simulink model "TosAeb"  (R2021a)
settings: Solver=FixedStepDiscrete  StartTime=0.0  StopTime=inf  FixedStep=0.01  RelTol=1e-3
undefined symbols (not defined in the file or in its folder): ACCModSts, AccSts1, AEB_ObjectMovingOrientation, AEB_ObjectMovingState, CN_BTCActive, CN_CurrentGearR, CN_CurrentGearR_DHT, CN_PTCActive, CN_VDCActive, CN_VSC_EspOff, CN_VSC_FCW_AEB_Error, CN_VSC_FCW_AEB_NoDispay, CN_VSC_FCW_AEB_PerfmDgdtn, CN_VSC_FCW_AEB_TempUnAvlbl, CN_VSC_VehStandstill, CNaccelAvoidMax, CNaccelAvoidMin, CNbrakePedalPress, CNcomfortStrLeftAccelMax, CNcomfortStrLeftAccelMin, CNcomfortStrRightAccelMax, CNcomfortStrRightAccelMin, CNDrvDoorOpen, CNDrvModeEco, CNDrvModeNorm, CNDrvModeOther, CNDrvModeSport, CNDrvSBRreminder, CNNull, CNobjInvalidID, CNPassDoorOpen, CNPassSBRreminder, CNPEBSAccelNegInf, CNPEBSAccelNull, CNPEBSTimeInf, CNPEBSTimeNull, CNSTOPMAX, CNSTOPNULL, CNsubjWidth, CNSwtOFF, CNSwtON, CNTTCMAX, CNTTCNULL, CNVehConfigAEBCycNone, CNVehConfigAEBNone, CNVehConfigAEBPedNone, CNVehConfigFCWNone, CNVehConfigJANone, CollisionSeverity, FALSE, FCWSnvty, JACycObjCalcInfoDefaultParams, JAPedObjCalcInfoDefaultParams, JAVehObjCalcInfoDefaultParams, latCycObjCalcInfoDefaultParams, latPedObjCalcInfoDefaultParams, longObjCalcInfoDefaultParams, ModSts, movingStatus, ObjectType ... (+1112)
libraries: simulink x3031, AEBSFunctionLib x50, Ta_Library x33, AEBSStateMachineLib x23, Mylibrary x12, simulink_extras x7, ObjSelect_lib x3, dspstat3 x2
(part 19 of 102)

== system TosAeb/FcwAebJa_Step_sys/ALG_AEBSystem/ALG_AEBController/AEB_VehicleStateCalcApp/VehMovingStateJudge/EngStateSuppFlg_HCU1 ==
  note: HCU_PowertrainSts:
      | 0x0:Sleep
      | 0x1:Initialize Mode
      | 0x2:power up
      | 0x3:power down
      | 0x4:Home
      | 0x5:run
      | 0x6:Charging
      | 0x7:Cellphone AC
      | 0x8:Limphome
      | 0x9:Emergency Mode
      | 0xA:V2L
      | 0xB:Prepare Sleep
      | 0xC:ready
      | 0xD:LV Charging
      | 0xE:LT Charging
      | 0xF:IL heating
      | 0x10:Thermal run away
      | 0x11:End of line
      | 0x12:BattTempKeep
      | 0x13-0x1F:Reserved
  note: HCU_Start_StopSts:
      | 0x0:non-start/stop mode
      | 0x1:Engine standby
      | 0x2:Normal operation
      | 0x3:Engine stop preparation
      | 0x4:Running down
      | 0x5:Engine stopped
      | 0x6:Cranking
      | 0x7:Starting
      | 0x8:Transmit torque
      | 0x9:Reserved
  note: EngState:
      | 0x0:Stopped
      | 0x1:Cranking
      | 0x2:Running
      | 0x3:Stalling
  EngState : Inport
  HCU_PowertrainSts : Inport  Port=2
  HCU_Start_StopSts : Inport  Port=3
  subjVel : Inport  Port=4
  Compare To Constant : simulink/Logic and Bit Operations/Compare To Constant  relop===  const=uint8(5)  OutDataTypeStr=boolean
  Compare To Constant1 : simulink/Logic and Bit Operations/Compare To Constant  relop===  const=uint8(5)  OutDataTypeStr=boolean
  Compare To Constant2 : simulink/Logic and Bit Operations/Compare To Constant  relop===  const=uint8(0)  OutDataTypeStr=boolean
  Compare To Constant4 : simulink/Logic and Bit Operations/Compare To Constant  relop=<  const=P_AEB_vehStateCalc_SubjVelThres4HCU_EngCond  OutDataTypeStr=boolean
  Constant : Constant  Value=FALSE
  Constant1 : Constant  Value=TRUE
  Logical Operator : Logic  Inputs=3  AllPortsSameDT=off  OutDataTypeStr=boolean
  Logical Operator1 : Logic  Operator=OR  AllPortsSameDT=off  OutDataTypeStr=boolean
  Switch : Switch  Criteria=u2 ~= 0
  EngStateSuppFlg : Outport
  wires:
  subjVel/1 -> Compare To Constant4/1
  Logical Operator1/1 -> Switch/2
  Logical Operator/1 -> Logical Operator1/2
  Compare To Constant4/1 -> Logical Operator/3
  Compare To Constant2/1 -> Logical Operator/2
  Compare To Constant1/1 -> Logical Operator/1
  EngState/1 -> Compare To Constant2/1
  HCU_Start_StopSts/1 -> Compare To Constant1/1
  Constant1/1 -> Switch/3
  Compare To Constant/1 -> Logical Operator1/1
  Constant/1 -> Switch/1
  HCU_PowertrainSts/1 -> Compare To Constant/1
  Switch/1 -> EngStateSuppFlg/1

== system TosAeb/FcwAebJa_Step_sys/ALG_AEBSystem/ALG_AEBController/AEB_VehicleStateCalcApp/VehMovingStateJudge/Subsystem1 ==
  note: 0x0:Select lever in position "P"
      | 0x1:Reserved
      | 0x2:Reserved
      | 0x3:Reserved
      | 0x4:Reserved
      | 0x5:Select lever in position "D"
      | 0x6:Select lever in position "N"
      | 0x7:Select lever in position "R"
      | 0x08-0xD:Reserved
      | 0xE:Intermediate position
      | 0xF:Invalid
  note: P_Global_VehiclePowerType
      | 0-V71混动；1-V61混动；
  CURRENT_GEAR : Inport
  HCU_GearSts : Inport  Port=2
  Compare To Constant : simulink/Logic and Bit Operations/Compare To Constant  relop===  const=CN_CurrentGearR  OutDataTypeStr=boolean
  Compare To Constant1 : simulink/Logic and Bit Operations/Compare To Constant  relop===  const=CN_CurrentGearR_DHT  OutDataTypeStr=boolean
  Constant : Constant  Value=P_Global_VehiclePowerType
  Multiport Switch : MultiPortSwitch  DataPortOrder=Specify indices  DataPortIndices={1,0}
  TCU_OperatingStateSuppFlg : Outport
  wires:
  Compare To Constant1/1 -> Multiport Switch/2  "TCUOperatingStateSuppFlg"
  Compare To Constant/1 -> Multiport Switch/3  "TCUOperatingStateSuppFlg"
  Multiport Switch/1 -> TCU_OperatingStateSuppFlg/1
  HCU_GearSts/1 -> Compare To Constant1/1
  Constant/1 -> Multiport Switch/1
  CURRENT_GEAR/1 -> Compare To Constant/1

== system TosAeb/FcwAebJa_Step_sys/ALG_AEBSystem/ALG_AEBController/AEB_VehicleStateCalcApp/VehSuppAborCondtionCalc/ABASuppAborConditonCalc ==
  AEB_VehResp : Inport
  FCW_AEB_VehFctSts : Inport  Port=2
  vehUnstableStateFlg : Inport  Port=3
  vehAssociatedSysStateSuppCond : Inport  Port=4
  "vehConfigAEB " : Inport  Port=5
  blockTimeBrakeReady : Inport  Port=6
  Bus Creator : BusCreator  Inputs=6
  Bus Selector : BusSelector  OutputSignals=AEB_VehResp,FCW_AEB_VehFctSts,vehUnstableSts,vehAssociatedSysStsSuppFlg,VehicleConfigAEB,blockTimeBrakeReady
  Bus Selector1 : BusSelector  OutputSignals=AEB_VehResp,FCW_AEB_VehFctSts
  VehStateCalcABAAborCalc : Subsystem
  VehStateCalcABAtSuppCalc : Subsystem
  VSCvehABASuppCond : Outport
  VSCvehABAAborCond : Outport  Port=2
  wires:
  VehStateCalcABAAborCalc/1(VSCvehABAAborCond) -> VSCvehABAAborCond/1
  VehStateCalcABAtSuppCalc/1(VSCvehABASuppCond) -> VSCvehABASuppCond/1
  Bus Selector1/2 -> VehStateCalcABAAborCalc/2(FCW_AEB_VehFctSts)  "<FCW_AEB_VehFctSts>"
  Bus Selector1/1 -> VehStateCalcABAAborCalc/1(AEB_VehResp)  "<AEB_VehResp>"
  Bus Selector/6 -> VehStateCalcABAtSuppCalc/6(blockTimeBrakeReady)  "<blockTimeBrakeReady>"
  Bus Selector/5 -> VehStateCalcABAtSuppCalc/5("vehConfigAEB ")  "<VehicleConfigAEB>"
  Bus Selector/4 -> VehStateCalcABAtSuppCalc/4(vehAssociatedSysStateSuppCond)  "<vehAssociatedSysStsSuppFlg>"
  Bus Selector/3 -> VehStateCalcABAtSuppCalc/3(vehUnstableStateFlg)  "<vehUnstableSts>"
  Bus Selector/2 -> VehStateCalcABAtSuppCalc/2(FCW_AEB_VehFctSts)  "<FCW_AEB_VehFctSts>"
  Bus Selector/1 -> VehStateCalcABAtSuppCalc/1(AEB_VehResp)  "<AEB_VehResp>"
  Bus Creator/1 -> Bus Selector1/1, Bus Selector/1
  AEB_VehResp/1 -> Bus Creator/1
  FCW_AEB_VehFctSts/1 -> Bus Creator/2
  blockTimeBrakeReady/1 -> Bus Creator/6
  vehUnstableStateFlg/1 -> Bus Creator/3
  "vehConfigAEB "/1 -> Bus Creator/5
  vehAssociatedSysStateSuppCond/1 -> Bus Creator/4

== system TosAeb/FcwAebJa_Step_sys/ALG_AEBSystem/ALG_AEBController/AEB_VehicleStateCalcApp/VehSuppAborCondtionCalc/AEBfullSuppAborConditonCalc ==
  VSCvehAEB_VehResp : Inport
  FCW_AEB_VehFctSts : Inport  Port=2
  vehUnstableStateFlg : Inport  Port=3
  vehAssociatedSysStateSuppCond : Inport  Port=4
  "vehConfigAEB " : Inport  Port=5
  blockTimeBrake : Inport  Port=6
  Bus Creator : BusCreator  Inputs=6
  Bus Selector : BusSelector  OutputSignals=AEB_VehResp,FCW_AEB_VehFctSts,vehUnstableSts,vehAssociatedSysStsSuppFlg,VehicleConfigAEB,blockTimeBrake
  Bus Selector1 : BusSelector  OutputSignals=AEB_VehResp,FCW_AEB_VehFctSts,vehAssociatedSysStsSuppFlg,vehUnstableSts
  VehStateCalcAEBfullAborCalc : Subsystem
  VehStateCalcAEBfullSuppCalc : Subsystem
  VSCvehAEBfullSuppCond : Outport
  VSCvehAEBfullAborCond : Outport  Port=2
  wires:
  VehStateCalcAEBfullAborCalc/1(VSCvehAEBfullAborCond) -> VSCvehAEBfullAborCond/1
  VehStateCalcAEBfullSuppCalc/1(VSCvehAEBfullSuppCond) -> VSCvehAEBfullSuppCond/1
  Bus Selector1/4 -> VehStateCalcAEBfullAborCalc/4(vehUnstableStateFlg)  "<vehUnstableSts>"
  Bus Selector1/3 -> VehStateCalcAEBfullAborCalc/3(vehAssociatedSysStateSuppCond)  "<vehAssociatedSysStsSuppFlg>"
  Bus Selector1/2 -> VehStateCalcAEBfullAborCalc/2(FCW_AEB_VehFctSts)  "<FCW_AEB_VehFctSts>"
  Bus Selector1/1 -> VehStateCalcAEBfullAborCalc/1(AEB_VehResp)  "<AEB_VehResp>"
  Bus Selector/6 -> VehStateCalcAEBfullSuppCalc/6(blockTimeBrake)  "<blockTimeBrake>"
  Bus Selector/5 -> VehStateCalcAEBfullSuppCalc/5("vehConfigAEB ")  "<VehicleConfigAEB>"
  Bus Selector/4 -> VehStateCalcAEBfullSuppCalc/4(vehAssociatedSysStateSuppCond)  "<vehAssociatedSysStsSuppFlg>"
  Bus Selector/3 -> VehStateCalcAEBfullSuppCalc/3(vehUnstableStateFlg)  "<vehUnstableSts>"
  Bus Selector/2 -> VehStateCalcAEBfullSuppCalc/2(FCW_AEB_VehFctSts)  "<FCW_AEB_VehFctSts>"
  Bus Selector/1 -> VehStateCalcAEBfullSuppCalc/1(AEB_VehResp)  "<AEB_VehResp>"
  Bus Creator/1 -> Bus Selector/1, Bus Selector1/1
  VSCvehAEB_VehResp/1 -> Bus Creator/1
  blockTimeBrake/1 -> Bus Creator/6
  FCW_AEB_VehFctSts/1 -> Bus Creator/2
  "vehConfigAEB "/1 -> Bus Creator/5
  vehUnstableStateFlg/1 -> Bus Creator/3
  vehAssociatedSysStateSuppCond/1 -> Bus Creator/4

== system TosAeb/FcwAebJa_Step_sys/ALG_AEBSystem/ALG_AEBController/AEB_VehicleStateCalcApp/VehSuppAborCondtionCalc/AEBpartSuppAborConditonCalc ==
  AEB_VehResp : Inport
  FCW_AEB_VehFctSts : Inport  Port=2
  vehUnstableStateFlg : Inport  Port=3
  vehAssociatedSysStateSuppCond : Inport  Port=4
  "vehConfigAEB " : Inport  Port=5
  blockTimeBrake : Inport  Port=6
  Bus Creator : BusCreator  Inputs=6
  Bus Selector : BusSelector  OutputSignals=AEB_VehResp,FCW_AEB_VehFctSts,vehUnstableSts,vehAssociatedSysStsSuppFlg,VehicleConfigAEB,blockTimeBrake
  Bus Selector1 : BusSelector  OutputSignals=AEB_VehResp,FCW_AEB_VehFctSts,vehAssociatedSysStsSuppFlg,vehUnstableSts
  VehStateCalcAEBpartAborCalc : Subsystem
  VehStateCalcAEBpartSuppCalc : Subsystem
  VSCvehAEBpartSuppCond : Outport
  VSCvehAEBpartAborCond : Outport  Port=2
  wires:
  VehStateCalcAEBpartAborCalc/1(VSCvehAEBpartAborCond) -> VSCvehAEBpartAborCond/1
  VehStateCalcAEBpartSuppCalc/1(VSCvehAEBpartSuppCond) -> VSCvehAEBpartSuppCond/1
  Bus Selector1/4 -> VehStateCalcAEBpartAborCalc/4(vehUnstableStateFlg)  "<vehUnstableSts>"
  Bus Selector1/3 -> VehStateCalcAEBpartAborCalc/3(vehAssociatedSysStateSuppCond)  "<vehAssociatedSysStsSuppFlg>"
  Bus Selector1/2 -> VehStateCalcAEBpartAborCalc/2(FCW_AEB_VehFctSts)  "<FCW_AEB_VehFctSts>"
  Bus Selector1/1 -> VehStateCalcAEBpartAborCalc/1(AEB_VehResp)  "<AEB_VehResp>"
  Bus Selector/1 -> VehStateCalcAEBpartSuppCalc/1(AEB_VehResp)  "<AEB_VehResp>"
  Bus Selector/6 -> VehStateCalcAEBpartSuppCalc/6(blockTimeBrake)  "<blockTimeBrake>"
  Bus Selector/2 -> VehStateCalcAEBpartSuppCalc/2(FCW_AEB_VehFctSts)  "<FCW_AEB_VehFctSts>"
  Bus Selector/5 -> VehStateCalcAEBpartSuppCalc/5("vehConfigAEB ")  "<VehicleConfigAEB>"
  Bus Selector/3 -> VehStateCalcAEBpartSuppCalc/3(vehUnstableStateFlg)  "<vehUnstableSts>"
  Bus Selector/4 -> VehStateCalcAEBpartSuppCalc/4(vehAssociatedSysStateSuppCond)  "<vehAssociatedSysStsSuppFlg>"
  Bus Creator/1 -> Bus Selector/1, Bus Selector1/1
  blockTimeBrake/1 -> Bus Creator/6
  "vehConfigAEB "/1 -> Bus Creator/5
  vehAssociatedSysStateSuppCond/1 -> Bus Creator/4
  vehUnstableStateFlg/1 -> Bus Creator/3
  FCW_AEB_VehFctSts/1 -> Bus Creator/2
  AEB_VehResp/1 -> Bus Creator/1

== system TosAeb/FcwAebJa_Step_sys/ALG_AEBSystem/ALG_AEBController/AEB_VehicleStateCalcApp/VehSuppAborCondtionCalc/AcuteWarningSuppAborConditonCalc ==
  AEB_VehResp : Inport
  FCW_AEB_VehFctSts : Inport  Port=2
  vehUnstableStateFlg : Inport  Port=3
  vehAssociatedSysStateSuppCond : Inport  Port=4
  "vehConfigFCW " : Inport  Port=5
  blockTimeWarning : Inport  Port=6
  Bus Creator : BusCreator  Inputs=6
  Bus Selector : BusSelector  OutputSignals=AEB_VehResp,FCW_AEB_VehFctSts,vehUnstableSts,vehAssociatedSysStsSuppFlg,VehicleConfigFCW,blockTimeWarning
  Bus Selector1 : BusSelector  OutputSignals=AEB_VehResp,FCW_AEB_VehFctSts,vehAssociatedSysStsSuppFlg
  VehStateCalcAcuteWarningAborCalc : Subsystem
  VehStateCalcAcuteWarningSuppCalc : Subsystem
  VSCvehAcuteWarningSuppCond : Outport
  VSCvehAcuteWarningAborCond : Outport  Port=2
  wires:
  VehStateCalcAcuteWarningAborCalc/1(VSCvehAcuteWarningAborCond) -> VSCvehAcuteWarningAborCond/1
  VehStateCalcAcuteWarningSuppCalc/1(VSCvehAcuteWarningSuppCond) -> VSCvehAcuteWarningSuppCond/1
  Bus Selector1/3 -> VehStateCalcAcuteWarningAborCalc/3(vehAssociatedSysStateSuppCond)  "<vehAssociatedSysStsSuppFlg>"
  Bus Selector1/1 -> VehStateCalcAcuteWarningAborCalc/1(AEB_VehResp)  "<AEB_VehResp>"
  Bus Selector1/2 -> VehStateCalcAcuteWarningAborCalc/2(FCW_AEB_VehFctSts)  "<FCW_AEB_VehFctSts>"
  Bus Selector/1 -> VehStateCalcAcuteWarningSuppCalc/1(AEB_VehResp)  "<AEB_VehResp>"
  Bus Selector/6 -> VehStateCalcAcuteWarningSuppCalc/6(blockTimeWarning)  "<blockTimeWarning>"
  Bus Selector/2 -> VehStateCalcAcuteWarningSuppCalc/2(FCW_AEB_VehFctSts)  "<FCW_AEB_VehFctSts>"
  Bus Selector/5 -> VehStateCalcAcuteWarningSuppCalc/5("vehConfigFCW ")  "<VehicleConfigFCW>"
  Bus Selector/3 -> VehStateCalcAcuteWarningSuppCalc/3(vehUnstableStateFlg)  "<vehUnstableSts>"
  Bus Selector/4 -> VehStateCalcAcuteWarningSuppCalc/4(vehAssociatedSysStateSuppCond)  "<vehAssociatedSysStsSuppFlg>"
  Bus Creator/1 -> Bus Selector1/1, Bus Selector/1
  AEB_VehResp/1 -> Bus Creator/1
  FCW_AEB_VehFctSts/1 -> Bus Creator/2
  vehUnstableStateFlg/1 -> Bus Creator/3
  vehAssociatedSysStateSuppCond/1 -> Bus Creator/4
  "vehConfigFCW "/1 -> Bus Creator/5
  blockTimeWarning/1 -> Bus Creator/6

== system TosAeb/FcwAebJa_Step_sys/ALG_AEBSystem/ALG_AEBController/AEB_VehicleStateCalcApp/VehSuppAborCondtionCalc/EBASuppAborConditonCalc ==
  AEB_VehResp : Inport
  FCW_AEB_VehFctSts : Inport  Port=2
  vehUnstableStateFlg : Inport  Port=3
  vehAssociatedSysStateSuppCond : Inport  Port=4
  "vehConfigAEB " : Inport  Port=5
  blockTimeBrake : Inport  Port=6
  Bus Creator : BusCreator  Inputs=6
  Bus Selector : BusSelector  OutputSignals=AEB_VehResp,FCW_AEB_VehFctSts,vehUnstableSts,vehAssociatedSysStsSuppFlg,VehicleConfigAEB,blockTimeBrake
  Bus Selector1 : BusSelector  OutputSignals=AEB_VehResp,FCW_AEB_VehFctSts,vehAssociatedSysStsSuppFlg
  VehStateCalcEBAAborCalc : Subsystem
  VehStateCalcEBASuppCalc : Subsystem
  VSCvehEBASuppCond : Outport
  VSCvehEBAAborCond : Outport  Port=2
  wires:
  VehStateCalcEBAAborCalc/1(VSCvehEBAAborCond) -> VSCvehEBAAborCond/1
  VehStateCalcEBASuppCalc/1(VSCvehEBASuppCond) -> VSCvehEBASuppCond/1
  Bus Selector1/3 -> VehStateCalcEBAAborCalc/3(vehAssociatedSysStateSuppCond)  "<vehAssociatedSysStsSuppFlg>"
  Bus Selector1/1 -> VehStateCalcEBAAborCalc/1(AEB_VehResp)  "<AEB_VehResp>"
  Bus Selector1/2 -> VehStateCalcEBAAborCalc/2(FCW_AEB_VehFctSts)  "<FCW_AEB_VehFctSts>"
  Bus Selector/1 -> VehStateCalcEBASuppCalc/1(AEB_VehResp)  "<AEB_VehResp>"
  Bus Selector/6 -> VehStateCalcEBASuppCalc/6(blockTimeBrake)  "<blockTimeBrake>"
  Bus Selector/2 -> VehStateCalcEBASuppCalc/2(FCW_AEB_VehFctSts)  "<FCW_AEB_VehFctSts>"
  Bus Selector/5 -> VehStateCalcEBASuppCalc/5("vehConfigAEB ")  "<VehicleConfigAEB>"
  Bus Selector/3 -> VehStateCalcEBASuppCalc/3(vehUnstableStateFlg)  "<vehUnstableSts>"
  Bus Selector/4 -> VehStateCalcEBASuppCalc/4(vehAssociatedSysStateSuppCond)  "<vehAssociatedSysStsSuppFlg>"
  Bus Creator/1 -> Bus Selector1/1, Bus Selector/1
  AEB_VehResp/1 -> Bus Creator/1
  FCW_AEB_VehFctSts/1 -> Bus Creator/2
  blockTimeBrake/1 -> Bus Creator/6
  vehUnstableStateFlg/1 -> Bus Creator/3
  "vehConfigAEB "/1 -> Bus Creator/5
  vehAssociatedSysStateSuppCond/1 -> Bus Creator/4

== system TosAeb/FcwAebJa_Step_sys/ALG_AEBSystem/ALG_AEBController/AEB_VehicleStateCalcApp/VehSuppAborCondtionCalc/LatentWarningSuppAborConditonCalc ==
  AEB_VehResp : Inport
  FCW_AEB_VehFctSts : Inport  Port=2
  vehUnstableStateFlg : Inport  Port=3
  vehAssociatedSysStateSuppCond : Inport  Port=4
  "vehConfigFCW " : Inport  Port=5
  blockTimeWarning : Inport  Port=6
  Bus Creator : BusCreator  Inputs=6
  Bus Selector : BusSelector  OutputSignals=AEB_VehResp,FCW_AEB_VehFctSts,vehUnstableSts,vehAssociatedSysStsSuppFlg,VehicleConfigFCW,blockTimeWarning
  Bus Selector1 : BusSelector  OutputSignals=AEB_VehResp,FCW_AEB_VehFctSts,vehAssociatedSysStsSuppFlg
  VehStateCalcLatentWarningAborCalc : Subsystem
  VehStateCalcLatentWarningSuppCalc : Subsystem
  VSCvehLatentWarningSuppCond : Outport
  VSCvehLatentWarningAborCond : Outport  Port=2
  wires:
  VehStateCalcLatentWarningAborCalc/1(VSCvehLatentWarningAborCond) -> VSCvehLatentWarningAborCond/1
  VehStateCalcLatentWarningSuppCalc/1(VSCvehLatentWarningSuppCond) -> VSCvehLatentWarningSuppCond/1
  Bus Selector1/3 -> VehStateCalcLatentWarningAborCalc/3(vehAssociatedSysStateSuppCond)  "<vehAssociatedSysStsSuppFlg>"
  Bus Selector1/1 -> VehStateCalcLatentWarningAborCalc/1(AEB_VehResp)  "<AEB_VehResp>"
  Bus Selector1/2 -> VehStateCalcLatentWarningAborCalc/2(FCW_AEB_VehFctSts)  "<FCW_AEB_VehFctSts>"
  Bus Selector/1 -> VehStateCalcLatentWarningSuppCalc/1(AEB_VehResp)  "<AEB_VehResp>"
  Bus Selector/6 -> VehStateCalcLatentWarningSuppCalc/6(blockTimeWarning)  "<blockTimeWarning>"
  Bus Selector/2 -> VehStateCalcLatentWarningSuppCalc/2(FCW_AEB_VehFctSts)  "<FCW_AEB_VehFctSts>"
  Bus Selector/5 -> VehStateCalcLatentWarningSuppCalc/5("vehConfigFCW ")  "<VehicleConfigFCW>"
  Bus Selector/3 -> VehStateCalcLatentWarningSuppCalc/3(vehUnstableStateFlg)  "<vehUnstableSts>"
  Bus Selector/4 -> VehStateCalcLatentWarningSuppCalc/4(vehAssociatedSysStateSuppCond)  "<vehAssociatedSysStsSuppFlg>"
  Bus Creator/1 -> Bus Selector1/1, Bus Selector/1
  blockTimeWarning/1 -> Bus Creator/6
  "vehConfigFCW "/1 -> Bus Creator/5
  vehAssociatedSysStateSuppCond/1 -> Bus Creator/4
  vehUnstableStateFlg/1 -> Bus Creator/3
  FCW_AEB_VehFctSts/1 -> Bus Creator/2
  AEB_VehResp/1 -> Bus Creator/1

== system TosAeb/FcwAebJa_Step_sys/ALG_AEBSystem/ALG_AEBController/AEB_VehicleStateCalcApp/VehSuppAborCondtionCalc/PreWarningSuppAborConditonCalc ==
  AEB_VehResp : Inport
  FCW_AEB_VehFctSts : Inport  Port=2
  vehUnstableStateFlg : Inport  Port=3
  vehAssociatedSysSuppStateFlg : Inport  Port=4
  "vehConfigFCW " : Inport  Port=5
  blockTimeWarning : Inport  Port=6
  Bus Creator : BusCreator  Inputs=6
  Bus Selector : BusSelector  OutputSignals=AEB_VehResp,FCW_AEB_VehFctSts,vehUnstableSts,vehAssociatedSysStsSuppFlg,VehicleConfigFCW,blockTimeWarning
  Bus Selector1 : BusSelector  OutputSignals=AEB_VehResp,FCW_AEB_VehFctSts,vehAssociatedSysStsSuppFlg
  VehStateCalcPreWarningAborCalc : Subsystem
  VehStateCalcPreWarningSuppCalc : Subsystem
  VSCvehPreWarningSuppCond : Outport
  VSCvehPreWarningAborCond : Outport  Port=2
  wires:
  VehStateCalcPreWarningAborCalc/1(VSCvehPreWarningAborCond) -> VSCvehPreWarningAborCond/1
  VehStateCalcPreWarningSuppCalc/1(VSCvehPreWarningSuppCond) -> VSCvehPreWarningSuppCond/1
  Bus Selector1/3 -> VehStateCalcPreWarningAborCalc/3(vehAssociatedSysStateSuppCond)  "<vehAssociatedSysStsSuppFlg>"
  Bus Selector1/2 -> VehStateCalcPreWarningAborCalc/2(FCW_AEB_VehFctSts)  "<FCW_AEB_VehFctSts>"
  Bus Selector1/1 -> VehStateCalcPreWarningAborCalc/1(AEB_VehResp)  "<AEB_VehResp>"
  Bus Selector/1 -> VehStateCalcPreWarningSuppCalc/1(AEB_VehResp)  "<AEB_VehResp>"
  Bus Selector/6 -> VehStateCalcPreWarningSuppCalc/6(blockTimeWarning)  "<blockTimeWarning>"
  Bus Selector/2 -> VehStateCalcPreWarningSuppCalc/2(FCW_AEB_VehFctSts)  "<FCW_AEB_VehFctSts>"
  Bus Selector/5 -> VehStateCalcPreWarningSuppCalc/5("vehConfigFCW ")  "<VehicleConfigFCW>"
  Bus Selector/3 -> VehStateCalcPreWarningSuppCalc/3(vehUnstableStateFlg)  "<vehUnstableSts>"
  Bus Selector/4 -> VehStateCalcPreWarningSuppCalc/4(vehAssociatedSysStateSuppCond)  "<vehAssociatedSysStsSuppFlg>"
  Bus Creator/1 -> Bus Selector1/1, Bus Selector/1
  AEB_VehResp/1 -> Bus Creator/1
  FCW_AEB_VehFctSts/1 -> Bus Creator/2
  vehUnstableStateFlg/1 -> Bus Creator/3
  vehAssociatedSysSuppStateFlg/1 -> Bus Creator/4
  blockTimeWarning/1 -> Bus Creator/6
  "vehConfigFCW "/1 -> Bus Creator/5

== system TosAeb/FcwAebJa_Step_sys/ALG_AEBSystem/ALG_AEBController/AEB_VehicleStateCalcApp/VehSuppAborCondtionCalc/PrefillSuppAborConditonCalc ==
  AEB_VehResp : Inport
  FCW_AEB_VehFctSts : Inport  Port=2
  vehUnstableStateFlg : Inport  Port=3
  vehAssociatedSysStateSuppCond : Inport  Port=4
  "vehConfigAEB " : Inport  Port=5
  blockTimeBrakeReady : Inport  Port=6
  Bus Creator : BusCreator  Inputs=6
  Bus Selector : BusSelector  OutputSignals=AEB_VehResp,FCW_AEB_VehFctSts,vehUnstableSts,vehAssociatedSysStsSuppFlg,VehicleConfigAEB,blockTimeBrakeReady
  Bus Selector1 : BusSelector  OutputSignals=AEB_VehResp,FCW_AEB_VehFctSts,vehUnstableSts,vehAssociatedSysStsSuppFlg
  VehStateCalcPrefillAborCalc : Subsystem
  VehStateCalcPrefillSuppCalc : Subsystem
  VSCvehPrefillSuppCond : Outport
  VSCvehPrefillAborCond : Outport  Port=2
  wires:
  VehStateCalcPrefillAborCalc/1(VSCvehPrefillAborCond) -> VSCvehPrefillAborCond/1
  VehStateCalcPrefillSuppCalc/1(VSCvehPrefillSuppCond) -> VSCvehPrefillSuppCond/1
  Bus Selector1/4 -> VehStateCalcPrefillAborCalc/4(vehAssociatedSysStateSuppCond)  "<vehAssociatedSysStsSuppFlg>"
  Bus Selector1/3 -> VehStateCalcPrefillAborCalc/3(vehUnstableStateFlg)  "<vehUnstableSts>"
  Bus Selector1/2 -> VehStateCalcPrefillAborCalc/2(FCW_AEB_VehFctSts)  "<FCW_AEB_VehFctSts>"
  Bus Selector1/1 -> VehStateCalcPrefillAborCalc/1(AEB_VehResp)  "<AEB_VehResp>"
  Bus Selector/6 -> VehStateCalcPrefillSuppCalc/6(blockTimeBrakeReady)  "<blockTimeBrakeReady>"
  Bus Selector/1 -> VehStateCalcPrefillSuppCalc/1(AEB_VehResp)  "<AEB_VehResp>"
  Bus Selector/5 -> VehStateCalcPrefillSuppCalc/5("vehConfigAEB ")  "<VehicleConfigAEB>"
  Bus Selector/2 -> VehStateCalcPrefillSuppCalc/2(FCW_AEB_VehFctSts)  "<FCW_AEB_VehFctSts>"
  Bus Selector/4 -> VehStateCalcPrefillSuppCalc/4(vehAssociatedSysStateSuppCond)  "<vehAssociatedSysStsSuppFlg>"
  Bus Selector/3 -> VehStateCalcPrefillSuppCalc/3(vehUnstableStateFlg)  "<vehUnstableSts>"
  Bus Creator/1 -> Bus Selector/1, Bus Selector1/1
  AEB_VehResp/1 -> Bus Creator/1
  blockTimeBrakeReady/1 -> Bus Creator/6
  FCW_AEB_VehFctSts/1 -> Bus Creator/2
  "vehConfigAEB "/1 -> Bus Creator/5
  vehUnstableStateFlg/1 -> Bus Creator/3
  vehAssociatedSysStateSuppCond/1 -> Bus Creator/4

== system TosAeb/FcwAebJa_Step_sys/ALG_AEBSystem/ALG_AEBInput/JaObjInfoMap/JaObjInfoMap/MovingStatus_Subsystem ==
  Alg_MovingStatus : Inport
  InputMovingStatus : Subsystem  TreatAsAtomicUnit=on
  MovingStatusSwitch : Stateflow chart  TreatAsAtomicUnit=on
  MovingStatusOut : Outport
  wires:
  Alg_MovingStatus/1 -> InputMovingStatus/1(MovingStatus)
  MovingStatusSwitch/1(MovingStatus) -> MovingStatusOut/1
  InputMovingStatus/1(Input_Unknown) -> MovingStatusSwitch/1(Input_Unknown)
  InputMovingStatus/2(Input_Stationary) -> MovingStatusSwitch/2(Input_Stationary)
  InputMovingStatus/3(Input_Stopped) -> MovingStatusSwitch/3(Input_Stopped)
  InputMovingStatus/4(Input_Moving) -> MovingStatusSwitch/4(Input_Moving)

== system TosAeb/FcwAebJa_Step_sys/ALG_AEBSystem/ALG_AEBInput/JaObjInfoMap/JaObjInfoMap/ObjType_Subsystem ==
  Alg_ObjType : Inport
  InputObjectType : Subsystem  TreatAsAtomicUnit=on
  ObjectType : Stateflow chart  TreatAsAtomicUnit=on
  ObjType : Outport
  wires:
  InputObjectType/4(Input_ObjMotor) -> ObjectType/4(ObjMotor)
  InputObjectType/6(Input_ObjGeneral) -> ObjectType/6(ObjGeneral)
  InputObjectType/3(Input_ObjPed) -> ObjectType/3(ObjPed)
  InputObjectType/7(Input_Unknown) -> ObjectType/7(Unknown)
  InputObjectType/5(Input_ObjBicycle) -> ObjectType/5(ObjBicycle)
  InputObjectType/2(Input_ObjTruck) -> ObjectType/2(ObjTruck)
  InputObjectType/1(Input_ObjCar) -> ObjectType/1(ObjCar)
  ObjectType/1(ObjType) -> ObjType/1
  Alg_ObjType/1 -> InputObjectType/1(u)

== system TosAeb/FcwAebJa_Step_sys/ALG_AEBSystem/ALG_AEBInput/JaObjInfoMap/JaObjInfoMap/Subsystem ==
  FR_ObjectType : Inport
  FR_ObjectMovingState : Inport  Port=2
  FR_ObjectMovingOrientation : Inport  Port=3
  Data Type Conversion : DataTypeConversion  OutDataTypeStr=Enum: AEB_ObjectMovingOrientation
  Data Type Conversion1 : DataTypeConversion  OutDataTypeStr=Enum: ObjectTypeCopy
  Data Type Conversion2 : DataTypeConversion  OutDataTypeStr=Enum: AEB_ObjectMovingState
  MovingStatus_Subsystem : Subsystem
  ObjType_Subsystem1 : Subsystem
  FR_ObjectTypeFilt : Outport
  FR_ObjectMovingStateFilt : Outport  Port=2
  FR_ObjectMovingOrientationFilt : Outport  Port=3
  wires:
  FR_ObjectType/1 -> ObjType_Subsystem1/1(Alg_ObjType)
  ObjType_Subsystem1/1(ObjType) -> Data Type Conversion1/1  "ObjectType"
  Data Type Conversion1/1 -> FR_ObjectTypeFilt/1  "ObjectType"
  MovingStatus_Subsystem/1(MovingStatusOut) -> Data Type Conversion2/1
  FR_ObjectMovingState/1 -> MovingStatus_Subsystem/1(Alg_MovingStatus)
  Data Type Conversion2/1 -> FR_ObjectMovingStateFilt/1
  FR_ObjectMovingOrientation/1 -> Data Type Conversion/1
  Data Type Conversion/1 -> FR_ObjectMovingOrientationFilt/1  "ObjectMovingOrientation"

== system TosAeb/FcwAebJa_Step_sys/ALG_AEBSystem/ALG_AEBInput/JaObjInfoMap/JaObjInfoMap/Subsystem1 ==
  FC_ObjectType : Inport
  FC_ObjectMovingState : Inport  Port=2
  FC_ObjectMovingOrientation : Inport  Port=3
  Data Type Conversion : DataTypeConversion  OutDataTypeStr=Enum: AEB_ObjectMovingOrientation
  Data Type Conversion1 : DataTypeConversion  OutDataTypeStr=Enum: ObjectTypeCopy
  Data Type Conversion2 : DataTypeConversion  OutDataTypeStr=Enum: AEB_ObjectMovingState
  MovingStatus_Subsystem : Subsystem
  ObjType_Subsystem1 : Subsystem
  FC_ObjectTypeFilt : Outport
  FC_ObjectMovingStateFilt : Outport  Port=2
  FC_ObjectMovingOrientationFilt : Outport  Port=3
  wires:
  FC_ObjectType/1 -> ObjType_Subsystem1/1(Alg_ObjType)
  ObjType_Subsystem1/1(ObjType) -> Data Type Conversion1/1  "ObjectType"
  Data Type Conversion1/1 -> FC_ObjectTypeFilt/1  "ObjectType"
  MovingStatus_Subsystem/1(MovingStatusOut) -> Data Type Conversion2/1
  FC_ObjectMovingState/1 -> MovingStatus_Subsystem/1(Alg_MovingStatus)
  Data Type Conversion2/1 -> FC_ObjectMovingStateFilt/1
  FC_ObjectMovingOrientation/1 -> Data Type Conversion/1
  Data Type Conversion/1 -> FC_ObjectMovingOrientationFilt/1  "ObjectMovingOrientation"

== system TosAeb/FcwAebJa_Step_sys/ALG_AEBSystem/ALG_AEBInput/LatObjInfoMap/latObjInfoMap/MovingStatus_Subsystem ==
  Alg_MovingStatus : Inport
  InputMovingStatus : Subsystem  TreatAsAtomicUnit=on
  MovingStatusSwitch : Stateflow chart  TreatAsAtomicUnit=on
  MovingStatusOut : Outport
  wires:
  Alg_MovingStatus/1 -> InputMovingStatus/1(MovingStatus)
  MovingStatusSwitch/1(MovingStatus) -> MovingStatusOut/1
  InputMovingStatus/1(Input_Unknown) -> MovingStatusSwitch/1(Input_Unknown)
  InputMovingStatus/2(Input_Stationary) -> MovingStatusSwitch/2(Input_Stationary)
  InputMovingStatus/3(Input_Stopped) -> MovingStatusSwitch/3(Input_Stopped)
  InputMovingStatus/4(Input_Moving) -> MovingStatusSwitch/4(Input_Moving)

== system TosAeb/FcwAebJa_Step_sys/ALG_AEBSystem/ALG_AEBInput/LatObjInfoMap/latObjInfoMap/ObjType_Subsystem ==
  Alg_ObjType : Inport
  InputObjectType : Subsystem  TreatAsAtomicUnit=on
  ObjectType : Stateflow chart  TreatAsAtomicUnit=on
  ObjType : Outport
  wires:
  InputObjectType/4(Input_ObjMotor) -> ObjectType/4(ObjMotor)
  InputObjectType/6(Input_ObjGeneral) -> ObjectType/6(ObjGeneral)
  InputObjectType/3(Input_ObjPed) -> ObjectType/3(ObjPed)
  InputObjectType/7(Input_Unknown) -> ObjectType/7(Unknown)
  InputObjectType/5(Input_ObjBicycle) -> ObjectType/5(ObjBicycle)
  InputObjectType/2(Input_ObjTruck) -> ObjectType/2(ObjTruck)
  InputObjectType/1(Input_ObjCar) -> ObjectType/1(ObjCar)
  ObjectType/1(ObjType) -> ObjType/1
  Alg_ObjType/1 -> InputObjectType/1(u)

== system TosAeb/FcwAebJa_Step_sys/ALG_AEBSystem/ALG_AEBInput/LatObjInfoMap/latObjInfoMap/Subsystem ==
  FR_ObjectType : Inport
  FR_ObjectMovingState : Inport  Port=2
  FR_ObjectMovingOrientation : Inport  Port=3
  Data Type Conversion : DataTypeConversion  OutDataTypeStr=Enum: AEB_ObjectMovingOrientation
  Data Type Conversion1 : DataTypeConversion  OutDataTypeStr=Enum: ObjectTypeCopy
  Data Type Conversion2 : DataTypeConversion  OutDataTypeStr=Enum: AEB_ObjectMovingState
  MovingStatus_Subsystem : Subsystem
  ObjType_Subsystem1 : Subsystem
  FR_ObjectTypeFilt : Outport
  FR_ObjectMovingStateFilt : Outport  Port=2
  FR_ObjectMovingOrientationFilt : Outport  Port=3
  wires:
  FR_ObjectType/1 -> ObjType_Subsystem1/1(Alg_ObjType)
  ObjType_Subsystem1/1(ObjType) -> Data Type Conversion1/1  "ObjectType"
  Data Type Conversion1/1 -> FR_ObjectTypeFilt/1  "ObjectType"
  MovingStatus_Subsystem/1(MovingStatusOut) -> Data Type Conversion2/1
  FR_ObjectMovingState/1 -> MovingStatus_Subsystem/1(Alg_MovingStatus)
  Data Type Conversion2/1 -> FR_ObjectMovingStateFilt/1
  FR_ObjectMovingOrientation/1 -> Data Type Conversion/1
  Data Type Conversion/1 -> FR_ObjectMovingOrientationFilt/1  "ObjectMovingOrientation"

== system TosAeb/FcwAebJa_Step_sys/ALG_AEBSystem/ALG_AEBInput/LatObjInfoMap/latObjInfoMap/Subsystem1 ==
  FC_ObjectType : Inport
  FC_ObjectMovingState : Inport  Port=2
  FC_ObjectMovingOrientation : Inport  Port=3
  Data Type Conversion : DataTypeConversion  OutDataTypeStr=Enum: AEB_ObjectMovingOrientation
  Data Type Conversion1 : DataTypeConversion  OutDataTypeStr=Enum: ObjectTypeCopy
  Data Type Conversion2 : DataTypeConversion  OutDataTypeStr=Enum: AEB_ObjectMovingState
  MovingStatus_Subsystem : Subsystem
  ObjType_Subsystem1 : Subsystem
  FC_ObjectTypeFilt : Outport
  FC_ObjectMovingStateFilt : Outport  Port=2
  FC_ObjectMovingOrientationFilt : Outport  Port=3
  wires:
  FC_ObjectType/1 -> ObjType_Subsystem1/1(Alg_ObjType)
  ObjType_Subsystem1/1(ObjType) -> Data Type Conversion1/1  "ObjectType"
  Data Type Conversion1/1 -> FC_ObjectTypeFilt/1
  MovingStatus_Subsystem/1(MovingStatusOut) -> Data Type Conversion2/1
  FC_ObjectMovingState/1 -> MovingStatus_Subsystem/1(Alg_MovingStatus)
  Data Type Conversion2/1 -> FC_ObjectMovingStateFilt/1
  Data Type Conversion/1 -> FC_ObjectMovingOrientationFilt/1  "ObjectMovingOrientation"
  FC_ObjectMovingOrientation/1 -> Data Type Conversion/1

== system TosAeb/FcwAebJa_Step_sys/ALG_AEBSystem/ALG_AEBInput/LongObjInfoMap/MovingStatus_Subsystem/InputMovingStatus ==
  note: 输出数值为3，类型为Moving
  note: 输出数值为2，类型为Stopped
  note: 输出数值为1，类型为Stationary
  note: 输出数值为0，类型为Unknown
  MovingStatus : Inport
  Compare To Constant : simulink/Logic and Bit Operations/Compare To Constant  relop===  const=0  OutDataTypeStr=boolean
  Compare To Constant1 : simulink/Logic and Bit Operations/Compare To Constant  relop===  const=1  OutDataTypeStr=boolean
  Compare To Constant2 : simulink/Logic and Bit Operations/Compare To Constant  relop===  const=3  OutDataTypeStr=boolean
  Compare To Constant8 : simulink/Logic and Bit Operations/Compare To Constant  relop===  const=2  OutDataTypeStr=boolean
  Input_Unknown : Outport
  Input_Stationary : Outport  Port=2
  Input_Stopped : Outport  Port=3
  Input_Moving : Outport  Port=4
  wires:
  Compare To Constant8/1 -> Input_Moving/1
  Compare To Constant1/1 -> Input_Stationary/1
  Compare To Constant2/1 -> Input_Stopped/1
  MovingStatus/1 -> Compare To Constant/1, Compare To Constant1/1, Compare To Constant2/1, Compare To Constant8/1
  Compare To Constant/1 -> Input_Unknown/1

== system TosAeb/FcwAebJa_Step_sys/ALG_AEBSystem/ALG_AEBInput/LongObjInfoMap/ObjType_Subsystem/InputObjectType ==
  note: 输出数值为2，类型为car
  note: 输出数值为3，类型为truck
  note: 输出数值为6，类型为pedestrian行人
  note: 输出数值为4，类型为motorbike摩托车
  note: 输出数值为5，类型为bicycle自行车
  note: 输出数值为7，类型为general_object
  note: 输出数值为1，类型为unknown
  InputObjType : Inport
  Compare To Constant : simulink/Logic and Bit Operations/Compare To Constant  relop===  const=0  OutDataTypeStr=boolean
  Compare To Constant1 : simulink/Logic and Bit Operations/Compare To Constant  relop===  const=1  OutDataTypeStr=boolean
  Compare To Constant10 : simulink/Logic and Bit Operations/Compare To Constant  relop===  const=7  OutDataTypeStr=boolean
  Compare To Constant11 : simulink/Logic and Bit Operations/Compare To Constant  relop===  const=9  OutDataTypeStr=boolean
  Compare To Constant12 : simulink/Logic and Bit Operations/Compare To Constant  relop===  const=8  OutDataTypeStr=boolean
  Compare To Constant13 : simulink/Logic and Bit Operations/Compare To Constant  relop===  const=15  OutDataTypeStr=boolean
  Compare To Constant14 : simulink/Logic and Bit Operations/Compare To Constant  relop===  const=10  OutDataTypeStr=boolean
  Compare To Constant15 : simulink/Logic and Bit Operations/Compare To Constant  relop===  const=11  OutDataTypeStr=boolean
  Compare To Constant16 : simulink/Logic and Bit Operations/Compare To Constant  relop===  const=12  OutDataTypeStr=boolean
  Compare To Constant17 : simulink/Logic and Bit Operations/Compare To Constant  relop===  const=14  OutDataTypeStr=boolean
  Compare To Constant18 : simulink/Logic and Bit Operations/Compare To Constant  relop===  const=12  OutDataTypeStr=boolean
  Compare To Constant2 : simulink/Logic and Bit Operations/Compare To Constant  relop===  const=16  OutDataTypeStr=boolean
  Compare To Constant3 : simulink/Logic and Bit Operations/Compare To Constant  relop===  const=2  OutDataTypeStr=boolean
  Compare To Constant4 : simulink/Logic and Bit Operations/Compare To Constant  relop===  const=3  OutDataTypeStr=boolean
  Compare To Constant5 : simulink/Logic and Bit Operations/Compare To Constant  relop===  const=4  OutDataTypeStr=boolean
  Compare To Constant6 : simulink/Logic and Bit Operations/Compare To Constant  relop===  const=5  OutDataTypeStr=boolean
  Compare To Constant7 : simulink/Logic and Bit Operations/Compare To Constant  relop===  const=18  OutDataTypeStr=boolean
  Compare To Constant8 : simulink/Logic and Bit Operations/Compare To Constant  relop===  const=17  OutDataTypeStr=boolean
  Compare To Constant9 : simulink/Logic and Bit Operations/Compare To Constant  relop===  const=6  OutDataTypeStr=boolean
  Logical Operator : Logic  Operator=OR  Inputs=4  AllPortsSameDT=off  OutDataTypeStr=boolean
  Logical Operator1 : Logic  Operator=OR  Inputs=5  AllPortsSameDT=off  OutDataTypeStr=boolean
  Logical Operator2 : Logic  Operator=OR  AllPortsSameDT=off  OutDataTypeStr=boolean
  Logical Operator3 : Logic  Operator=OR  AllPortsSameDT=off  OutDataTypeStr=boolean
  Logical Operator5 : Logic  Operator=OR  Inputs=4  AllPortsSameDT=off  OutDataTypeStr=boolean
  Input_ObjCar : Outport
  Input_ObjTruck : Outport  Port=2
  Input_ObjPed : Outport  Port=3
  Input_ObjMotor : Outport  Port=4
  Input_ObjBicycle : Outport  Port=5
  Input_ObjGeneral : Outport  Port=6
  Input_Unknown : Outport  Port=7
  wires:
  Compare To Constant12/1 -> Logical Operator3/1
  Compare To Constant6/1 -> Logical Operator1/4
  Logical Operator/1 -> Input_ObjCar/1
  Compare To Constant13/1 -> Logical Operator3/2
  InputObjType/1 -> Compare To Constant8/1, Compare To Constant18/1, Compare To Constant17/1, Compare To Constant16/1, Compare To Constant15/1, Compare To Constant14/1, Compare To Constant13/1, Compare To Constant12/1, Compare To Constant11/1, Compare To Constant10/1, Compare To Constant9/1, Compare To Constant7/1, Compare To Constant6/1, Compare To Constant5/1, Compare To Constant4/1, Compare To Constant3/1, Compare To Constant2/1, Compare To Constant1/1, Compare To Constant/1
  Compare To Constant2/1 -> Logical Operator/3
  Compare To Constant1/1 -> Logical Operator/2
  Compare To Constant16/1 -> Logical Operator5/3
  Compare To Constant18/1 -> Input_Unknown/1
  Compare To Constant15/1 -> Logical Operator5/2
  Compare To Constant10/1 -> Logical Operator2/1
  Logical Operator5/1 -> Input_ObjGeneral/1
  Compare To Constant5/1 -> Logical Operator1/3
  Logical Operator3/1 -> Input_ObjBicycle/1
  Logical Operator1/1 -> Input_ObjTruck/1
  Compare To Constant14/1 -> Logical Operator5/1
  Compare To Constant3/1 -> Logical Operator1/1
  Logical Operator2/1 -> Input_ObjMotor/1
  Compare To Constant7/1 -> Logical Operator1/5
  Compare To Constant4/1 -> Logical Operator1/2
  Compare To Constant11/1 -> Logical Operator2/2
  Compare To Constant8/1 -> Logical Operator/4
  Compare To Constant/1 -> Logical Operator/1
  Compare To Constant17/1 -> Logical Operator5/4
  Compare To Constant9/1 -> Input_ObjPed/1

== system TosAeb/FcwAebJa_Step_sys/ALG_AEBSystem/ALG_AEBInput/LongObjInfoMap/Subsystem/MovingStatus_Subsystem ==
  Alg_MovingStatus : Inport
  InputMovingStatus : Subsystem  TreatAsAtomicUnit=on
  MovingStatusSwitch : Stateflow chart  TreatAsAtomicUnit=on
  MovingStatusOut : Outport
  wires:
  Alg_MovingStatus/1 -> InputMovingStatus/1(MovingStatus)
  MovingStatusSwitch/1(MovingStatus) -> MovingStatusOut/1
  InputMovingStatus/1(Input_Unknown) -> MovingStatusSwitch/1(Input_Unknown)
  InputMovingStatus/2(Input_Stationary) -> MovingStatusSwitch/2(Input_Stationary)
  InputMovingStatus/3(Input_Stopped) -> MovingStatusSwitch/3(Input_Stopped)
  InputMovingStatus/4(Input_Moving) -> MovingStatusSwitch/4(Input_Moving)

== system TosAeb/FcwAebJa_Step_sys/ALG_AEBSystem/ALG_AEBInput/LongObjInfoMap/Subsystem/ObjType_Subsystem ==
  Alg_ObjType : Inport
  InputObjectType : Subsystem  TreatAsAtomicUnit=on
  ObjectType : Stateflow chart  TreatAsAtomicUnit=on
  ObjType : Outport
  wires:
  InputObjectType/4(Input_ObjMotor) -> ObjectType/4(ObjMotor)
  InputObjectType/6(Input_ObjGeneral) -> ObjectType/6(ObjGeneral)
  InputObjectType/3(Input_ObjPed) -> ObjectType/3(ObjPed)
  InputObjectType/7(Input_Unknown) -> ObjectType/7(Unknown)
  InputObjectType/5(Input_ObjBicycle) -> ObjectType/5(ObjBicycle)
  InputObjectType/2(Input_ObjTruck) -> ObjectType/2(ObjTruck)
  InputObjectType/1(Input_ObjCar) -> ObjectType/1(ObjCar)
  ObjectType/1(ObjType) -> ObjType/1
  Alg_ObjType/1 -> InputObjectType/1(InputObjType)

== system TosAeb/FcwAebJa_Step_sys/ALG_AEBSystem/ALG_AEBInput/LongObjInfoMap/Subsystem1/MovingStatus_Subsystem ==
  Alg_MovingStatus : Inport
  InputMovingStatus : Subsystem  TreatAsAtomicUnit=on
  MovingStatusSwitch : Stateflow chart  TreatAsAtomicUnit=on
  MovingStatusOut : Outport
  wires:
  Alg_MovingStatus/1 -> InputMovingStatus/1(MovingStatus)
  MovingStatusSwitch/1(MovingStatus) -> MovingStatusOut/1
  InputMovingStatus/1(Input_Unknown) -> MovingStatusSwitch/1(Input_Unknown)
  InputMovingStatus/2(Input_Stationary) -> MovingStatusSwitch/2(Input_Stationary)
  InputMovingStatus/3(Input_Stopped) -> MovingStatusSwitch/3(Input_Stopped)
  InputMovingStatus/4(Input_Moving) -> MovingStatusSwitch/4(Input_Moving)

== system TosAeb/FcwAebJa_Step_sys/ALG_AEBSystem/ALG_AEBInput/LongObjInfoMap/Subsystem1/ObjType_Subsystem1 ==
  Alg_ObjType : Inport
  InputObjectType : Subsystem  TreatAsAtomicUnit=on
  ObjectType : Stateflow chart  TreatAsAtomicUnit=on
  ObjType : Outport
  wires:
  InputObjectType/4(Input_ObjMotor) -> ObjectType/4(ObjMotor)
  InputObjectType/6(Input_ObjGeneral) -> ObjectType/6(ObjGeneral)
  InputObjectType/3(Input_ObjPed) -> ObjectType/3(ObjPed)
  InputObjectType/7(Input_Unknown) -> ObjectType/7(Unknown)
  InputObjectType/5(Input_ObjBicycle) -> ObjectType/5(ObjBicycle)
  InputObjectType/2(Input_ObjTruck) -> ObjectType/2(ObjTruck)
  InputObjectType/1(Input_ObjCar) -> ObjectType/1(ObjCar)
  ObjectType/1(ObjType) -> ObjType/1
  Alg_ObjType/1 -> InputObjectType/1(InputObjType)

== system TosAeb/FcwAebJa_Step_sys/ALG_AEBSystem/ALG_AEBOutput/AEBS_DVRInfoCalc/AEB_JAPED_DVR_INFO/If Action Subsystem ==
  Action Port : ActionPort  ActionPortLabel=if(u1)
  Bus Creator : BusCreator  Inputs=12
  Constant{,1..11} (12 alike) : Constant  Value=0
  Data Type Conversion{,1..11} (12 alike) : DataTypeConversion
  AEB_JAPED_DVR_INFO : Outport
  wires:
  Constant10/1 -> Data Type Conversion10/1
  Constant11/1 -> Data Type Conversion11/1
  Constant9/1 -> Data Type Conversion9/1
  Constant8/1 -> Data Type Conversion8/1
  Constant4/1 -> Data Type Conversion4/1
  Constant5/1 -> Data Type Conversion5/1
  Constant7/1 -> Data Type Conversion7/1
  Constant6/1 -> Data Type Conversion6/1
  Constant2/1 -> Data Type Conversion2/1
  Constant3/1 -> Data Type Conversion3/1
  Constant1/1 -> Data Type Conversion1/1
  Constant/1 -> Data Type Conversion/1
  Data Type Conversion3/1 -> Bus Creator/4  "JAPedObjDx"
  Data Type Conversion4/1 -> Bus Creator/5  "JAPedObjDy"
  Data Type Conversion/1 -> Bus Creator/1  "JAPedObjID"
  Data Type Conversion6/1 -> Bus Creator/7  "JAPedObjVy"
  Data Type Conversion9/1 -> Bus Creator/10  "JAPedObjExist"
  Data Type Conversion7/1 -> Bus Creator/8  "JAPedObjAx"
  Data Type Conversion10/1 -> Bus Creator/11  "JAPedObjObstcl"
  Data Type Conversion2/1 -> Bus Creator/3  "JAPedObjState"
  Data Type Conversion11/1 -> Bus Creator/12  "JAPedObjCntAlive"
  Data Type Conversion5/1 -> Bus Creator/6  "JAPedObjVx"
  Bus Creator/1 -> AEB_JAPED_DVR_INFO/1
  Data Type Conversion1/1 -> Bus Creator/2  "JAPedObjVideoCfmd"
  Data Type Conversion8/1 -> Bus Creator/9  "JAPedObjAy"

== system TosAeb/FcwAebJa_Step_sys/ALG_AEBSystem/ALG_AEBOutput/AEBS_DVRInfoCalc/AEB_JAPED_DVR_INFO/Subsystem ==
  JAPedTgtCalcInfo : Inport
  Action Port : ActionPort  ActionPortLabel=else
  AEBObjStateMap4 : Subsystem
  Bus Creator : BusCreator  Inputs=12
  Bus Selector4 : BusSelector  OutputSignals=JAPedTgtObjInfo.AEBJAPedObj.ObjectID,JAPedTgtObjInfo.AEBJAPedObj.ObjectTypeCameraConfirmed,JAPedTgtObjInfo.AEBJAPedObj.ObjectMovingState,JAPedTgtObjInfo.AEBJAPedObj.ObjectMovingOrientation,JAPedTgtObjInfo.AEBJAPedObj.dx,JAPedTgtObjInfo.AEBJAPedObj.dy,JAPedTgtObjInfo.AEBJAPedObj.vx,JAPedTgtObjInfo.AEBJAPedObj.vy,JAPedTgtObjInfo.AEBJAPedObj.ax,JAPedTgtObjInfo.AEBJAPedObj.ay,JAPedTgtObjInfo.AEBJAPedObj.ObjectExistProb,JAPedTgtObjInfo.AEBJAPedObj.ObjectIsObstacleProb,JAPedTgtObjInfo.AEBJAPedObj.ObjectLifeCycle
  Signal Conversion{50..61} (12 alike) : SignalConversion  OverrideOpt=off
  AEB_JAPED_DVR_INFO : Outport
  wires:
  Signal Conversion55/1 -> Bus Creator/6  "JAPedObjVx"
  Signal Conversion61/1 -> Bus Creator/12  "JAPedObjCntAlive"
  Signal Conversion59/1 -> Bus Creator/10  "JAPedObjExist"
  Signal Conversion57/1 -> Bus Creator/8  "JAPedObjAx"
  Signal Conversion52/1 -> Bus Creator/3  "JAPedObjState"
  Signal Conversion53/1 -> Bus Creator/4  "JAPedObjDx"
  Signal Conversion60/1 -> Bus Creator/11  "JAPedObjObstcl"
  Bus Selector4/4 -> AEBObjStateMap4/2(ObjectMovingOrientation)  "<ObjectMovingOrientation>"
  Signal Conversion56/1 -> Bus Creator/7  "JAPedObjVy"
  Bus Selector4/3 -> AEBObjStateMap4/1(ObjectMovingState)  "<ObjectMovingState>"
  Bus Selector4/11 -> Signal Conversion59/1  "<ObjectExistProb>"
  Bus Selector4/8 -> Signal Conversion56/1  "<vy>"
  Bus Selector4/7 -> Signal Conversion55/1  "<vx>"
  AEBObjStateMap4/1(AEBObjState) -> Signal Conversion52/1
  Signal Conversion50/1 -> Bus Creator/1  "JAPedObjID"
  Bus Selector4/1 -> Signal Conversion50/1  "<ObjectID>"
  Signal Conversion54/1 -> Bus Creator/5  "JAPedObjDy"
  Bus Selector4/10 -> Signal Conversion58/1  "<ay>"
  Bus Creator/1 -> AEB_JAPED_DVR_INFO/1
  Bus Selector4/2 -> Signal Conversion51/1  "<ObjectTypeCameraConfirmed>"
  Bus Selector4/12 -> Signal Conversion60/1  "<ObjectIsObstacleProb>"
  Bus Selector4/5 -> Signal Conversion53/1  "<dx>"
  Signal Conversion58/1 -> Bus Creator/9  "JAPedObjAy"
  Bus Selector4/13 -> Signal Conversion61/1  "<ObjectLifeCycle>"
  Signal Conversion51/1 -> Bus Creator/2  "JAPedObjVideoCfmd"
  Bus Selector4/6 -> Signal Conversion54/1  "<dy>"
  JAPedTgtCalcInfo/1 -> Bus Selector4/1
  Bus Selector4/9 -> Signal Conversion57/1  "<ax>"

== system TosAeb/FcwAebJa_Step_sys/ALG_AEBSystem/ALG_AEBOutput/AEBS_DVRInfoCalc/AEB_JAVEH_DVR_INFO/If Action Subsystem ==
  Action Port : ActionPort  ActionPortLabel=if(u1)
  Bus Creator : BusCreator  Inputs=12
  Constant{,1..11} (12 alike) : Constant  Value=0
  Data Type Conversion{,1..11} (12 alike) : DataTypeConversion
  AEB_JAVEH_DVR_INFO : Outport
  wires:
  Constant6/1 -> Data Type Conversion6/1
  Constant7/1 -> Data Type Conversion7/1
  Constant8/1 -> Data Type Conversion8/1
  Constant11/1 -> Data Type Conversion11/1
  Constant10/1 -> Data Type Conversion10/1
  Constant9/1 -> Data Type Conversion9/1
  Constant3/1 -> Data Type Conversion3/1
  Constant4/1 -> Data Type Conversion4/1
  Constant5/1 -> Data Type Conversion5/1
  Constant2/1 -> Data Type Conversion2/1
  Constant1/1 -> Data Type Conversion1/1
  Constant/1 -> Data Type Conversion/1
  Data Type Conversion9/1 -> Bus Creator/10  "AEBJAObjExist"
  Data Type Conversion7/1 -> Bus Creator/8  "AEBJAObjAx"
  Data Type Conversion3/1 -> Bus Creator/4  "AEBJAObjDx"
  Data Type Conversion5/1 -> Bus Creator/6  "AEBJAObjVx"
  Data Type Conversion2/1 -> Bus Creator/3  "AEBJAObjState"
  Data Type Conversion4/1 -> Bus Creator/5  "AEBJAObjDy"
  Data Type Conversion6/1 -> Bus Creator/7  "AEBJAObjVy"
  Data Type Conversion1/1 -> Bus Creator/2  "AEBJAObjVideoCfmd"
  Data Type Conversion8/1 -> Bus Creator/9  "AEBJAObjAy"
  Data Type Conversion/1 -> Bus Creator/1  "AEBJAObjID"
  Data Type Conversion10/1 -> Bus Creator/11  "AEBJAObjObstcl"
  Data Type Conversion11/1 -> Bus Creator/12  "AEBJAObjCntAlive"
  Bus Creator/1 -> AEB_JAVEH_DVR_INFO/1

== system TosAeb/FcwAebJa_Step_sys/ALG_AEBSystem/ALG_AEBOutput/AEBS_DVRInfoCalc/AEB_JAVEH_DVR_INFO/Subsystem ==
  JAVehTgtCalcInfo : Inport
  Action Port : ActionPort  ActionPortLabel=else
  AEBObjStateMap : Subsystem
  Bus Creator : BusCreator  Inputs=12
  Bus Selector3 : BusSelector  OutputSignals=JAVehTgtObjInfo.AEBJAVehObj.ObjectID,JAVehTgtObjInfo.AEBJAVehObj.ObjectTypeCameraConfirmed,JAVehTgtObjInfo.AEBJAVehObj.ObjectMovingState,JAVehTgtObjInfo.AEBJAVehObj.ObjectMovingOrientation,JAVehTgtObjInfo.AEBJAVehObj.dx,JAVehTgtObjInfo.AEBJAVehObj.dy,JAVehTgtObjInfo.AEBJAVehObj.vx,JAVehTgtObjInfo.AEBJAVehObj.vy,JAVehTgtObjInfo.AEBJAVehObj.ax,JAVehTgtObjInfo.AEBJAVehObj.ay,JAVehTgtObjInfo.AEBJAVehObj.ObjectExistProb,JAVehTgtObjInfo.AEBJAVehObj.ObjectIsObstacleProb,JAVehTgtObjInfo.AEBJAVehObj.ObjectLifeCycle
  Signal Conversion{38..49} (12 alike) : SignalConversion  OverrideOpt=off
  AEB_JAVEH_DVR_INFO : Outport
  wires:
  Bus Creator/1 -> AEB_JAVEH_DVR_INFO/1
  Signal Conversion49/1 -> Bus Creator/12  "AEBJAObjCntAlive"
  Signal Conversion48/1 -> Bus Creator/11  "AEBJAObjObstcl"
  Signal Conversion47/1 -> Bus Creator/10  "AEBJAObjExist"
  Signal Conversion46/1 -> Bus Creator/9  "AEBJAObjAy"
  Signal Conversion45/1 -> Bus Creator/8  "AEBJAObjAx"
  Signal Conversion38/1 -> Bus Creator/1  "AEBJAObjID"
  Signal Conversion44/1 -> Bus Creator/7  "AEBJAObjVy"
  Signal Conversion39/1 -> Bus Creator/2  "AEBJAObjVideoCfmd"
  Signal Conversion43/1 -> Bus Creator/6  "AEBJAObjVx"
  Signal Conversion40/1 -> Bus Creator/3  "AEBJAObjState"
  Signal Conversion42/1 -> Bus Creator/5  "AEBJAObjDy"
  Signal Conversion41/1 -> Bus Creator/4  "AEBJAObjDx"
  Bus Selector3/10 -> Signal Conversion46/1  "<ay>"
  Bus Selector3/12 -> Signal Conversion48/1  "<ObjectIsObstacleProb>"
  Bus Selector3/4 -> AEBObjStateMap/2(ObjectMovingOrientation)  "<ObjectMovingOrientation>"
  Bus Selector3/8 -> Signal Conversion44/1  "<vy>"
  Bus Selector3/7 -> Signal Conversion43/1  "<vx>"
  JAVehTgtCalcInfo/1 -> Bus Selector3/1
  Bus Selector3/3 -> AEBObjStateMap/1(ObjectMovingState)  "<ObjectMovingState>"
  Bus Selector3/1 -> Signal Conversion38/1  "<ObjectID>"
  Bus Selector3/13 -> Signal Conversion49/1  "<ObjectLifeCycle>"
  Bus Selector3/9 -> Signal Conversion45/1  "<ax>"
  AEBObjStateMap/1(AEBObjState) -> Signal Conversion40/1
  Bus Selector3/6 -> Signal Conversion42/1  "<dy>"
  Bus Selector3/5 -> Signal Conversion41/1  "<dx>"
  Bus Selector3/11 -> Signal Conversion47/1  "<ObjectExistProb>"
  Bus Selector3/2 -> Signal Conversion39/1  "<ObjectTypeCameraConfirmed>"

== system TosAeb/FcwAebJa_Step_sys/ALG_AEBSystem/ALG_AEBOutput/AEBS_DVRInfoCalc/AEB_LATCYC_DVR_INFO/If Action Subsystem ==
  Action Port : ActionPort  ActionPortLabel=if(u1)
  Bus Creator : BusCreator  Inputs=12
  Constant{,1..11} (12 alike) : Constant  Value=0
  Data Type Conversion{,1..11} (12 alike) : DataTypeConversion
  AEB_LATCYC_DVR_INFO : Outport
  wires:
  Constant6/1 -> Data Type Conversion6/1
  Constant7/1 -> Data Type Conversion7/1
  Constant8/1 -> Data Type Conversion8/1
  Constant11/1 -> Data Type Conversion11/1
  Constant10/1 -> Data Type Conversion10/1
  Constant9/1 -> Data Type Conversion9/1
  Constant3/1 -> Data Type Conversion3/1
  Constant4/1 -> Data Type Conversion4/1
  Constant5/1 -> Data Type Conversion5/1
  Constant2/1 -> Data Type Conversion2/1
  Constant1/1 -> Data Type Conversion1/1
  Constant/1 -> Data Type Conversion/1
  Data Type Conversion7/1 -> Bus Creator/8  "CyclistObjAx"
  Data Type Conversion9/1 -> Bus Creator/10  "CyclistObjExist"
  Data Type Conversion6/1 -> Bus Creator/7  "CyclistObjVy"
  Data Type Conversion3/1 -> Bus Creator/4  "CyclistObjDx"
  Data Type Conversion8/1 -> Bus Creator/9  "CyclistObjAy"
  Data Type Conversion10/1 -> Bus Creator/11  "CyclistObjObstcl"
  Data Type Conversion11/1 -> Bus Creator/12  "CyclistObjCntAlive"
  Data Type Conversion1/1 -> Bus Creator/2  "CyclistObjVideoCfmd"
  Data Type Conversion/1 -> Bus Creator/1  "CyclistObjID"
  Data Type Conversion5/1 -> Bus Creator/6  "CyclistObjVx"
  Data Type Conversion2/1 -> Bus Creator/3  "CyclistObjState"
  Data Type Conversion4/1 -> Bus Creator/5  "CyclistObjDy"
  Bus Creator/1 -> AEB_LATCYC_DVR_INFO/1
